simulink library "Renault_Library"  (Simulink 8.2)
settings: Solver=ode3  StartTime=0.0  StopTime=10.0  FixedStep=0.020  RelTol=1e-3
undefined symbols (not defined in the file or in its folder): ABC, AssystemProposal, b, chkval, CoeffA_C, CoeffB_C, CoeffMean_C, ConfTime_C, DiagDelay_C, DividePercent, FailureConfActive_C, FallingEdgeDuration_C, HighLevelThresholdVoltage_C, HighLevelVoltage_C, HighLevelVoltageTunable_C, InitValue_C, InputVoltRangeMax_C, InputVoltRangeMin_C, LowActiveContact_C, LowLevelThresholdVoltage_C, LowLevelVoltage_C, MaxOperatingVoltage_C, MinOperatingVoltage_C, mV2V_conversion, OUT1, OutOfRangeHigh_C, OutOfRangeLow_C, PinSupplySaturationMax, PinSupplySaturationMin, Res1Value_C, Res2Value_C, Res3Value_C, RisingEdgeDuration_C, RSA_CANSignalFailMonitoring, SampleTime_C, service14Table, service19Table, service22Table, service24Table, service28Table, service2ETable, service2FTable, service31Table, service87Table, SizeXStateDID2FTable, SizeXTable14, SizeXTable19, SizeXTable22, SizeXTable24, SizeXTable28, SizeXTable2E, SizeXTable2F, SizeXTable31, SizeXTable87, SizeYStateDID2FTable, SizeYTable14, SizeYTable19, SizeYTable22, SizeYTable24, SizeYTable28 ... (+22)
undefined symbols (not defined in the file): BatteryVoltage_ActivationThreshold_C, FrameStatus_AbsentValue_C, FrameStatus_ConfAbsentValue_C, MemThreshold_C, NAMEOFTHEMODEL_SAMPLE_TIME_CST, NbAbs1_C, NbConfAbs1_C, NbConfPres1_C, NbPres1_C, NOMDUSIGNAL_FaultConfTime_C, NOMDUSIGNAL_FaultRehabTime_C, NOMDUSIGNAL_switchvalue_C, NOMDUSIGNAL_unavailablevalue_C, PeriodFrame1_C, ResetTime_C, WakeUpTime_C
libraries: Renault_Library x112, simulink x75
(part 11 of 32)

== system Renault_Library/Complex blocks/Timers & Counters/RSA_Timer_v01_05 ==
  Init : Inport
      settings: AttributesFormatString=Type : %<OutDataTypeStr>
  Duration : Inport  Port=2
      settings: AttributesFormatString=Type : %<OutDataTypeStr>
  Constant1 : Constant  Tag=AM,Constant,0,1,Value;CMP,Value;  Value=TIM1INI  OutDataTypeStr=Inherit: Inherit via back propagation  SampleTime=-1
  Constant2 : Constant  Tag=AM,Constant,0,1,Value;CMP,Value;  Value=TIM1INI  OutDataTypeStr=Inherit: Inherit via back propagation  SampleTime=-1
  Constant3 : Constant  Tag=AM,Constant,0,1,Value;CMP,Value;  Value=st  OutDataTypeStr=Inherit: Inherit via back propagation  SampleTime=-1
  Constant4 : Constant  Tag=AM,Constant,0,1,Value;CMP,Value;  Value=st  OutDataTypeStr=Inherit: Inherit via back propagation  SampleTime=-1
  Constant5 : Constant  Tag=AM,Constant,0,1,Value;CMP,Value;  Value=1000  OutDataTypeStr=uint16  SampleTime=-1
  Constant6 : Constant  Tag=AM,Constant,0,1,Value;CMP,Value;  OutDataTypeStr=Inherit: Inherit via back propagation  SampleTime=-1
  DataTypeConversion : DataTypeConversion  RndMeth=Zero
  DataTypeConversion1 : DataTypeConversion  OutDataTypeStr=uint32  SaturateOnIntegerOverflow=on  RndMeth=Zero
  DataTypeConversion2 : DataTypeConversion  RndMeth=Zero
  DelayInput1 : UnitDelay  Description=\nStore in Global RAM  InitialCondition=timeoutinit  SampleTime=-1
      settings: AttributesFormatString=IC = %<X0>
  DelayInput2 : UnitDelay  Description=\nStore in Global RAM  InitialCondition=TIM1INI  SampleTime=-1
      settings: AttributesFormatString=IC = %<X0>
  EdgeRising : Subsystem  mask(EdgeRising){vinit=initinit}
      mask prompts: vinit "Initialisation"
      mask help: When a rising edge is used as input, the ouput is equal to one during the fall of the signal. Otherwise the output is zero.
      mask Display:
      | plot([0.2 0.4 0.4 0.6],[0.6 0.6 0.9 0.9]);
      | plot([0.2 0.4 0.4 0.4 0.6],[0.1 0.1 0.4 0.1 0.1])
  LogicalOperator : Logic  OutDataTypeStr=boolean
  Product : Product  SaturateOnIntegerOverflow=on  RndMeth=Zero
  Product1 : Product  SaturateOnIntegerOverflow=on  RndMeth=Zero
  Product2 : Product  Inputs=*/  OutDataTypeStr=Inherit: Inherit via back propagation  SaturateOnIntegerOverflow=on  RndMeth=Zero
  RSA_CompareToZero : Subsystem  Tag=[LIB]  mask(RSA_CompareToZero){relop=~=, LogicOutDataTypeMode=boolean, ZeroCross=on}
      mask prompts: relop "Operator:"; LogicOutDataTypeMode "Output data type mode:"; ZeroCross "Enable zero-crossing detection"
      mask help:
      | RSA_CompareToZero block compares an input signal to zero.
      | Specify how the input is compared to zero with the “Operator” parameter.
      | The “Operator” parameter can have the following values:
      |       1. "==" — Determine whether the input is equal to zero.
      |       2. "~=" — Determine whether the input is not equal to zero.
      |       3. "<"  — Determine whether the input is less than zero.
      |       4. "<=" — Determine whether the input is less than or equal to zero.
      |       5. ">"  — Determine whether the input is greater than zero.
      |       6. ">=" — Determine whether the input is greater than or equal to zero.
      |
      | The output is 0 if the comparison is false, and 1 if it is true.
      |
      | Version : 01.03
      mask init: same as Renault_Library/Complex blocks/Logic & Bit Operations/RSA_CompareToZero
      settings: AttributesFormatString=%<MaskType> : Ver : 01.03\nOperator: %<relop>\nOutput data type mode: %<LogicOutDataTypeMode>\nEnable zero-crossing detection %<ZeroCross>
      mask Help: eval(['open([','''\\F-khepri\shared\DIM-DCMAP-CGMP\66161\Shared_MatLab\Tools_DCMAP\Common_Library_MBDR\Library\Common_Library\Help\RLV_RSA_CompareToZero_Spec_V1.03.pdf''','])'])
      mask TypeOptions relop: == | ~= | < | <= | >= | >
      mask TypeOptions LogicOutDataTypeMode: uint8 | boolean
      mask Display: disp([relop,' 0'])
  RSA_DataTypeDuplicate_v01_03 : Subsystem  Tag=[LIB]  mask(RSA_DataTypeDuplicate){NoIn=2}
      mask prompts: NoIn "No Of Inputs"
      mask help:
      | Force all inputs to have the exact same data type.
      |
      | Version : 01.03
      mask init: same as Renault_Library/Complex blocks/Signal Routing/RSA_DataTypeDuplicate_v01_03
      settings: AttributesFormatString=%<MaskType> : Ver : 01.03\nNo Of Inputs %<NoIn>
      mask Help: eval(['open([','''\\F-khepri\shared\DIM-DCMAP-CGMP\66161\Shared_MatLab\Tools_DCMAP\Common_Library_MBDR\Library\Common_Library\Help\RLV_RSA_DataTypeDuplicate_Spec_V1.03.pdf''','])'])
      mask Display: disp('Same\n DT')
  RelationalOperator1 : RelationalOperator  Operator=>  OutDataTypeStr=boolean  ZeroCross=off  InputSameDT=on
  RelationalOperator2 : RelationalOperator  Operator=<=  OutDataTypeStr=boolean  ZeroCross=off  InputSameDT=on
  Subtract4 : Sum  Inputs=-+  OutDataTypeStr=Inherit: Inherit via back propagation  RndMeth=Zero  SaturateOnIntegerOverflow=on  InputSameDT=on
      settings: AttributesFormatString=SAT = %<SaturateOnIntegerOverflow>
  Sum : Sum  Inputs=+-  OutDataTypeStr=Inherit: Inherit via back propagation  RndMeth=Zero  SaturateOnIntegerOverflow=on  InputSameDT=on
      settings: AttributesFormatString=SAT = %<SaturateOnIntegerOverflow>
  Switch{1..5} (5 alike) : Switch  Tag=GEN,Threshold;AM,Switch,2,1;CMP,Threshold;  Criteria=u2 ~= 0  RndMeth=Zero  SaturateOnIntegerOverflow=on  InputSameDT=on
      settings: AttributesFormatString=Criteria=%<Criteria>
  Timeout : Outport
      settings: AttributesFormatString=Type : %<OutDataTypeStr>\nInit :%<InitialOutput>
  Timer : Outport  Port=2
      settings: AttributesFormatString=Type : %<OutDataTypeStr>\nInit :%<InitialOutput>
  wires:
  Constant2/1 -> Switch3/1
  Switch2/1 -> RelationalOperator1/2, Switch3/3  "X3"
  Constant1/1 -> Switch4/3
  Switch4/1 -> Switch2/3
  RelationalOperator2/1 -> Switch4/2
  DataTypeConversion/1 -> Timer/1
  EdgeRising/1(Out) -> Switch1/2, Switch1/1, [EdgeRising]
  Init/1 -> EdgeRising/1(In)
  LogicalOperator/1 -> Timeout/1
  Switch1/1 -> DelayInput1/1, LogicalOperator/1  "Flag_of_first_initialisation"
  DelayInput1/1 -> Switch1/3
  RelationalOperator1/1 -> Switch3/2, LogicalOperator/2  "X4"
  [Timestep1] -> Sum/2, RelationalOperator2/1
  [EdgeRising] -> Switch2/2
  DelayInput2/1 -> Sum/1, RelationalOperator2/2  "X2"
  Sum/1 -> Switch4/1
  Constant3/1 -> DataTypeConversion1/1, Subtract4/2
  DataTypeConversion1/1 -> DataTypeConversion2/1
  DataTypeConversion2/1 -> Subtract4/1
  RSA_CompareToZero/1(y) -> Switch5/2
  Subtract4/1 -> RSA_CompareToZero/1(u)
  Constant6/1 -> Switch5/3
  Constant5/1 -> Switch5/1
  Switch5/1 -> [tolcons]
  Product/1 -> [Timestep1]
  Constant4/1 -> Product/1, RSA_DataTypeDuplicate_v01_03/1(In1)
  [tolcons] -> Product/2
  Duration/1 -> RSA_DataTypeDuplicate_v01_03/2(In2), Product1/1
  [tolcons] -> Product1/2
  Product1/1 -> Switch2/1
  [Timestep1] -> RelationalOperator1/1
  Switch3/1 -> Product2/1, DelayInput2/1
  [tolcons] -> Product2/2
  Product2/1 -> DataTypeConversion/1

== system Renault_Library/Complex blocks/Timers & Counters/RSA_Timer_v01_06 ==
  Init : Inport
      settings: AttributesFormatString=Type : %<OutDataTypeStr>
  Duration : Inport  Port=2
      settings: AttributesFormatString=Type : %<OutDataTypeStr>
  Constant1 : Constant  Tag=AM,Constant,0,1,Value;CMP,Value;  Value=TIM1INI  OutDataTypeStr=Inherit: Inherit via back propagation  SampleTime=-1
  Constant2 : Constant  Tag=AM,Constant,0,1,Value;CMP,Value;  Value=TIM1INI  OutDataTypeStr=Inherit: Inherit via back propagation  SampleTime=-1
  Constant4 : Constant  Tag=AM,Constant,0,1,Value;CMP,Value;  Value=st  OutDataTypeStr=Inherit: Inherit via back propagation  SampleTime=-1
  Constant5 : Constant  Tag=AM,Constant,0,1,Value;CMP,Value;  Value=1000  OutDataTypeStr=uint16  SampleTime=-1
  Constant6 : Constant  Tag=AM,Constant,0,1,Value;CMP,Value;  OutDataTypeStr=Inherit: Inherit via back propagation  SampleTime=-1
  DataTypeConversion : DataTypeConversion  RndMeth=Zero
  DataTypeConversion1 : DataTypeConversion  OutDataTypeStr=uint32  SaturateOnIntegerOverflow=on  RndMeth=Zero
  DataTypeConversion2 : DataTypeConversion  RndMeth=Zero
  DelayInput1 : UnitDelay  Description=\nStore in Global RAM  InitialCondition=timeoutinit  SampleTime=-1
      settings: AttributesFormatString=IC = %<X0>
  DelayInput2 : UnitDelay  Description=\nStore in Global RAM  InitialCondition=TIM1INI  SampleTime=-1
      settings: AttributesFormatString=IC = %<X0>
  EdgeRising : Subsystem  mask(EdgeRising){vinit=initinit}
      mask prompts: vinit "Initialisation"
      mask help: When a rising edge is used as input, the ouput is equal to one during the fall of the signal. Otherwise the output is zero.
      mask Display:
      | plot([0.2 0.4 0.4 0.6],[0.6 0.6 0.9 0.9]);
      | plot([0.2 0.4 0.4 0.4 0.6],[0.1 0.1 0.4 0.1 0.1])
  LogicalOperator : Logic  OutDataTypeStr=boolean
  Product : Product  SaturateOnIntegerOverflow=on  RndMeth=Zero
  Product1 : Product  SaturateOnIntegerOverflow=on  RndMeth=Zero
  Product2 : Product  Inputs=*/  OutDataTypeStr=Inherit: Inherit via back propagation  SaturateOnIntegerOverflow=on  RndMeth=Zero
  RSA_CompareToZero : Renault_Library/Complex blocks/Logic & Bit Operations/RSA_CompareToZero  Tag=[LIB]  SystemSampleTime=-1  relop=~=  LogicOutDataTypeMode=boolean
      settings: AttributesFormatString=%<MaskType> : Ver : 01.03\nOperator: %<relop>\nOutput data type mode: %<LogicOutDataTypeMode>\nEnable zero-crossing detection %<ZeroCross>
  RSA_DataTypeDuplicate_v01_03 : Renault_Library/Complex blocks/Signal Routing/RSA_DataTypeDuplicate_v01_03  Tag=[LIB]  SystemSampleTime=-1  NoIn=2
      settings: AttributesFormatString=%<MaskType> : Ver : 01.03\nNo Of Inputs %<NoIn>
  RelationalOperator1 : RelationalOperator  Operator=>  OutDataTypeStr=boolean  ZeroCross=off  InputSameDT=on
  RelationalOperator2 : RelationalOperator  Operator=<=  OutDataTypeStr=boolean  ZeroCross=off  InputSameDT=on
  Subtract4 : Sum  Inputs=-+  OutDataTypeStr=Inherit: Inherit via back propagation  RndMeth=Zero  SaturateOnIntegerOverflow=on  InputSameDT=on
      settings: AttributesFormatString=SAT = %<SaturateOnIntegerOverflow>
  Sum : Sum  Inputs=+-  OutDataTypeStr=Inherit: Inherit via back propagation  RndMeth=Zero  SaturateOnIntegerOverflow=on  InputSameDT=on
      settings: AttributesFormatString=SAT = %<SaturateOnIntegerOverflow>
  Switch{1..5} (5 alike) : Switch  Tag=GEN,Threshold;AM,Switch,2,1;CMP,Threshold;  Criteria=u2 ~= 0  RndMeth=Zero  SaturateOnIntegerOverflow=on  InputSameDT=on
      settings: AttributesFormatString=Criteria=%<Criteria>
  Timeout : Outport
      settings: AttributesFormatString=Type : %<OutDataTypeStr>\nInit :%<InitialOutput>
  Timer : Outport  Port=2
      settings: AttributesFormatString=Type : %<OutDataTypeStr>\nInit :%<InitialOutput>
  wires:
  Constant2/1 -> Switch3/1
  Switch2/1 -> RelationalOperator1/2, Switch3/3  "X3"
  Constant1/1 -> Switch4/3
  Switch4/1 -> Switch2/3
  RelationalOperator2/1 -> Switch4/2
  DataTypeConversion/1 -> Timer/1
  EdgeRising/1(Out) -> Switch1/2, Switch1/1, [EdgeRising]
  Init/1 -> EdgeRising/1(In)
  LogicalOperator/1 -> Timeout/1
  Switch1/1 -> DelayInput1/1, LogicalOperator/1  "Flag_of_first_initialisation"
  DelayInput1/1 -> Switch1/3
  RelationalOperator1/1 -> Switch3/2, LogicalOperator/2  "X4"
  [Timestep1] -> Sum/2, RelationalOperator2/1
  [EdgeRising] -> Switch2/2
  DelayInput2/1 -> Sum/1, RelationalOperator2/2  "X2"
  Sum/1 -> Switch4/1
  [st] -> DataTypeConversion1/1, Subtract4/2
  DataTypeConversion1/1 -> DataTypeConversion2/1
  DataTypeConversion2/1 -> Subtract4/1
  RSA_CompareToZero/1 -> Switch5/2
  Subtract4/1 -> RSA_CompareToZero/1
  Constant6/1 -> Switch5/3
  Constant5/1 -> Switch5/1
  Switch5/1 -> [tolcons]
  Product/1 -> [Timestep1]
  Constant4/1 -> RSA_DataTypeDuplicate_v01_03/1, [st], Product/1
  [tolcons] -> Product/2
  Duration/1 -> RSA_DataTypeDuplicate_v01_03/2, Product1/1
  [tolcons] -> Product1/2
  Product1/1 -> Switch2/1
  [Timestep1] -> RelationalOperator1/1
  Switch3/1 -> Product2/1, DelayInput2/1
  [tolcons] -> Product2/2
  Product2/1 -> DataTypeConversion/1

== system Renault_Library/Complex blocks/Timers & Counters/RSA_Timer_v01_07 ==
  note: Flag_of_first_initialisation
  Init : Inport
      settings: AttributesFormatString=Type : %<OutDataTypeStr>
  Duration : Inport  Port=2
      settings: AttributesFormatString=Type : %<OutDataTypeStr>
  Constant1 : Constant  Tag=AM,Constant,0,1,Value;CMP,Value;  Value=TIM1INI  OutDataTypeStr=Inherit: Inherit via back propagation  SampleTime=-1
  Constant2 : Constant  Tag=AM,Constant,0,1,Value;CMP,Value;  Value=TIM1INI  OutDataTypeStr=Inherit: Inherit via back propagation  SampleTime=-1
  Constant3 : Constant  Tag=AM,Constant,0,1,Value;CMP,Value;  Value=st  OutDataTypeStr=SampleDT
  Constant5 : Constant  Value=Boosting_Factor  OutDataTypeStr=Constant_DT
  DelayInput1 : UnitDelay  Description=\nStore in Global RAM  InitialCondition=timeoutinit  SampleTime=-1
      settings: AttributesFormatString=IC = %<X0>
  DelayInput2 : UnitDelay  Description=\nStore in Global RAM  InitialCondition=TIM1INI  SampleTime=-1
      settings: AttributesFormatString=IC = %<X0>
  EdgeRising : Subsystem  mask(EdgeRising){vinit=initinit}
      mask prompts: vinit "Initialisation"
      mask help: When a rising edge is used as input, the ouput is equal to one during the fall of the signal. Otherwise the output is zero.
      mask Display:
      | plot([0.2 0.4 0.4 0.6],[0.6 0.6 0.9 0.9]);
      | plot([0.2 0.4 0.4 0.4 0.6],[0.1 0.1 0.4 0.1 0.1])
  LogicalOperator : Logic  OutDataTypeStr=boolean
  Product2 : Product  Inputs=*/  OutDataTypeStr=Inherit: Inherit via back propagation  SaturateOnIntegerOverflow=on  RndMeth=Zero
  Product3 : Product  OutDataTypeStr=Inherit: Inherit via back propagation  SaturateOnIntegerOverflow=on  RndMeth=Zero
  Product4 : Product  OutDataTypeStr=Inherit: Inherit via back propagation  SaturateOnIntegerOverflow=on  RndMeth=Zero
  RelationalOperator1 : RelationalOperator  Operator=>  OutDataTypeStr=boolean  ZeroCross=off  InputSameDT=on
  RelationalOperator2 : RelationalOperator  Operator=<=  OutDataTypeStr=boolean  ZeroCross=off  InputSameDT=on
  Sum : Sum  Inputs=+-  OutDataTypeStr=Inherit: Inherit via back propagation  RndMeth=Zero  InputSameDT=on
      settings: AttributesFormatString=SAT = %<SaturateOnIntegerOverflow>
  Switch{1..4} (4 alike) : Switch  Tag=GEN,Threshold;AM,Switch,2,1;CMP,Threshold;  Criteria=u2 ~= 0  RndMeth=Zero  SaturateOnIntegerOverflow=on  InputSameDT=on
      settings: AttributesFormatString=Criteria=%<Criteria>
  Timeout : Outport
      settings: AttributesFormatString=Type : %<OutDataTypeStr>\nInit :%<InitialOutput>
  Timer : Outport  Port=2
      settings: AttributesFormatString=Type : %<OutDataTypeStr>\nInit :%<InitialOutput>
  wires:
  Constant2/1 -> Switch3/1
  Switch2/1 -> RelationalOperator1/2, Switch3/3
  Constant1/1 -> Switch4/3
  Switch4/1 -> Switch2/3
  RelationalOperator2/1 -> Switch4/2
  EdgeRising/1(Out) -> Switch1/2, Switch1/1, [EdgeRising]
  Init/1 -> EdgeRising/1(In)
  LogicalOperator/1 -> Timeout/1
  Switch1/1 -> LogicalOperator/1, DelayInput1/1
  DelayInput1/1 -> Switch1/3
  RelationalOperator1/1 -> Switch3/2, LogicalOperator/2
  [Timestep1] -> Sum/2, RelationalOperator2/1
  [EdgeRising] -> Switch2/2
  DelayInput2/1 -> Sum/1, RelationalOperator2/2
  Sum/1 -> Switch4/1
  [Timestep1] -> RelationalOperator1/1
  Switch3/1 -> DelayInput2/1, Product2/1
  [tolcons] -> Product2/2
  Constant5/1 -> [tolcons]
  [tolcons] -> Product3/2
  [tolcons] -> Product4/2
  Product4/1 -> [dly]
  Product3/1 -> [Timestep1]
  Constant3/1 -> Product3/1
  Duration/1 -> Product4/1
  [dly] -> Switch2/1
  Product2/1 -> Timer/1

== system Renault_Library/Complex blocks/Timers & Counters/RSA_TurnOffDelay2 ==
  in : Inport
      settings: AttributesFormatString=Type : %<OutDataTypeStr>
  dly : Inport  Port=2
      settings: AttributesFormatString=Type : %<OutDataTypeStr>
  Constant1 : Constant  Value=INIVAL  OutDataTypeStr=Inherit: Inherit via back propagation  SampleTime=-1
  Constant2 : Constant  Value=Ts  OutDataTypeStr=Inherit: Inherit via back propagation  SampleTime=-1
  Logical_Operator1 : Logic  Operator=OR  OutDataTypeStr=boolean
  Logical_Operator2 : Logic  OutDataTypeStr=boolean
  Logical_Operator3 : Logic  Operator=NOT  OutDataTypeStr=boolean
  Logical_Operator4 : Logic  Operator=NOT  OutDataTypeStr=boolean
  MinMax : MinMax  Function=max  Inputs=2  OutDataTypeStr=Inherit: Inherit via back propagation  RndMeth=Zero  SaturateOnIntegerOverflow=on  InputSameDT=on
  Relational_Operator8 : RelationalOperator  Operator=<=  OutDataTypeStr=boolean  InputSameDT=on
  Sum1 : Sum  Inputs=+-  OutDataTypeStr=Inherit: Inherit via back propagation  RndMeth=Zero  SaturateOnIntegerOverflow=on  InputSameDT=on
      settings: AttributesFormatString=SAT = %<SaturateOnIntegerOverflow>
  Switch1 : Switch  Criteria=u2 ~= 0  RndMeth=Zero  SaturateOnIntegerOverflow=on  InputSameDT=on
      settings: AttributesFormatString=Criteria=%<Criteria>
  Switch2 : Switch  Criteria=u2 ~= 0  RndMeth=Zero  SaturateOnIntegerOverflow=on  InputSameDT=on
      settings: AttributesFormatString=Criteria=%<Criteria>
  UnitDelay : UnitDelay  InitialCondition=false  SampleTime=-1
  Unit_Delay2 : UnitDelay  InitialCondition=INIVAL  SampleTime=-1
  out : Outport
      settings: AttributesFormatString=Type : %<OutDataTypeStr>\nInit :%<InitialOutput>
  ctr : Outport  Port=2
      settings: AttributesFormatString=Type : %<OutDataTypeStr>\nInit :%<InitialOutput>
  wires:
  Constant1/1 -> [INIVAL], Switch1/1
  Constant2/1 -> Sum1/2
  [dly] -> Switch2/1
  [INIVAL] -> Relational_Operator8/2
  Unit_Delay2/1 -> Sum1/1
  Relational_Operator8/1 -> Logical_Operator4/1
  Logical_Operator1/1 -> out/1
  Sum1/1 -> MinMax/1
  MinMax/1 -> Switch2/3
  Switch2/1 -> Switch1/3
  Switch1/1 -> Unit_Delay2/1, ctr/1, Relational_Operator8/1
  UnitDelay/1 -> Logical_Operator2/2
  Logical_Operator2/1 -> Switch2/2
  Logical_Operator3/1 -> Logical_Operator2/1
  Logical_Operator4/1 -> Logical_Operator1/2
  dly/1 -> [dly]
  [INIVAL] -> MinMax/2
  in/1 -> UnitDelay/1, Logical_Operator3/1, Logical_Operator1/1, Switch1/2

== system Renault_Library/Complex blocks/Timers & Counters/RSA_TurnOffDelay2_v01_03 ==
  in : Inport
      settings: AttributesFormatString=Type : %<OutDataTypeStr>
  dly : Inport  Port=2
      settings: AttributesFormatString=Type : %<OutDataTypeStr>
  Constant1 : Constant  Value=INIVAL  OutDataTypeStr=Inherit: Inherit via back propagation  SampleTime=-1
  Constant2 : Constant  Value=Ts  OutDataTypeStr=Inherit: Inherit via back propagation  SampleTime=-1
  Constant3 : Constant  Value=Ts  OutDataTypeStr=Inherit: Inherit via back propagation  SampleTime=-1
  Logical_Operator1 : Logic  Operator=OR  OutDataTypeStr=boolean
  Logical_Operator2 : Logic  OutDataTypeStr=boolean
  Logical_Operator3 : Logic  Operator=NOT  OutDataTypeStr=boolean
  Relational_Operator1 : RelationalOperator  Operator=<=  OutDataTypeStr=boolean  InputSameDT=on
  Relational_Operator8 : RelationalOperator  Operator=>  OutDataTypeStr=boolean  InputSameDT=on
  Sum1 : Sum  Inputs=+-  OutDataTypeStr=Inherit: Inherit via back propagation  RndMeth=Zero  SaturateOnIntegerOverflow=on  InputSameDT=on
      settings: AttributesFormatString=SAT = %<SaturateOnIntegerOverflow>
  Switch1 : Switch  Criteria=u2 ~= 0  RndMeth=Zero  SaturateOnIntegerOverflow=on  InputSameDT=on
      settings: AttributesFormatString=Criteria=%<Criteria>
  Switch2 : Switch  Criteria=u2 ~= 0  RndMeth=Zero  SaturateOnIntegerOverflow=on  InputSameDT=on
      settings: AttributesFormatString=Criteria=%<Criteria>
  Switch3 : Switch  Criteria=u2 ~= 0  RndMeth=Zero  SaturateOnIntegerOverflow=on  InputSameDT=on
      settings: AttributesFormatString=Criteria=%<Criteria>
  UnitDelay : UnitDelay  InitialCondition=false  SampleTime=-1
      settings: AttributesFormatString=IC = %<X0>
  Unit_Delay2 : UnitDelay  InitialCondition=INIVAL  SampleTime=-1
      settings: AttributesFormatString=IC = %<X0>
  out : Outport
      settings: AttributesFormatString=Type : %<OutDataTypeStr>\nInit :%<InitialOutput>
  ctr : Outport  Port=2
      settings: AttributesFormatString=Type : %<OutDataTypeStr>\nInit :%<InitialOutput>
  wires:
  Constant1/1 -> Switch1/1, [INIVAL]
  Constant2/1 -> Sum1/2
  [dly] -> Switch2/1
  [INIVAL] -> Relational_Operator8/2
  Unit_Delay2/1 -> Relational_Operator1/1, Sum1/1
  Logical_Operator1/1 -> out/1
  Switch2/1 -> Switch1/3
  Switch1/1 -> Unit_Delay2/1, ctr/1, Relational_Operator8/1
  UnitDelay/1 -> Logical_Operator2/2
  Logical_Operator2/1 -> Switch2/2
  Logical_Operator3/1 -> Logical_Operator2/1
  dly/1 -> [dly]
  in/1 -> UnitDelay/1, Logical_Operator3/1, Logical_Operator1/1, Switch1/2
  Relational_Operator8/1 -> Logical_Operator1/2
  Switch3/1 -> Switch2/3
  Sum1/1 -> Switch3/3
  Relational_Operator1/1 -> Switch3/2
  [INIVAL] -> Switch3/1
  Constant3/1 -> Relational_Operator1/2

== system Renault_Library/Complex blocks/Timers & Counters/RSA_TurnOffDelay2_v01_05 ==
  in : Inport
      settings: AttributesFormatString=Type : %<OutDataTypeStr>
  dly : Inport  Port=2
      settings: AttributesFormatString=Type : %<OutDataTypeStr>
  Constant1 : Constant  Value=INIVAL  OutDataTypeStr=Inherit: Inherit via back propagation  SampleTime=-1
  Constant4 : Constant  Tag=AM,Constant,0,1,Value;CMP,Value;  Value=Ts  OutDataTypeStr=Inherit: Inherit via back propagation  SampleTime=-1
  Constant5 : Constant  Tag=AM,Constant,0,1,Value;CMP,Value;  Value=1000  OutDataTypeStr=uint16  SampleTime=-1
  Constant6 : Constant  Tag=AM,Constant,0,1,Value;CMP,Value;  OutDataTypeStr=Inherit: Inherit via back propagation  SampleTime=-1
  DataTypeConversion1 : DataTypeConversion  OutDataTypeStr=uint32  SaturateOnIntegerOverflow=on  RndMeth=Zero
  DataTypeConversion2 : DataTypeConversion  RndMeth=Zero
  DataTypeConversion3 : DataTypeConversion  RndMeth=Zero
  Logical_Operator1 : Logic  Operator=OR  OutDataTypeStr=boolean
  Logical_Operator2 : Logic  OutDataTypeStr=boolean
  Logical_Operator3 : Logic  Operator=NOT  OutDataTypeStr=boolean
  Product : Product  SaturateOnIntegerOverflow=on  RndMeth=Zero
  Product1 : Product  SaturateOnIntegerOverflow=on  RndMeth=Zero
  Product2 : Product  Inputs=*/  SaturateOnIntegerOverflow=on  RndMeth=Zero
  RSA_CompareToZero : Renault_Library/Complex blocks/Logic & Bit Operations/RSA_CompareToZero  Tag=[LIB]  SystemSampleTime=-1  relop===  LogicOutDataTypeMode=boolean
      settings: AttributesFormatString=%<MaskType> : Ver : 01.03\nOperator: %<relop>\nOutput data type mode: %<LogicOutDataTypeMode>\nEnable zero-crossing detection %<ZeroCross>
  RSA_DataTypeDuplicate_v01_03 : Renault_Library/Complex blocks/Signal Routing/RSA_DataTypeDuplicate_v01_03  Tag=[LIB]  SystemSampleTime=-1  NoIn=2
      settings: AttributesFormatString=%<MaskType> : Ver : 01.03\nNo Of Inputs %<NoIn>
  Relational_Operator1 : RelationalOperator  Operator=<=  OutDataTypeStr=boolean  InputSameDT=on
  Relational_Operator8 : RelationalOperator  OutDataTypeStr=boolean  InputSameDT=on
  Subtract4 : Sum  Inputs=-+  OutDataTypeStr=Inherit: Inherit via back propagation  RndMeth=Zero  SaturateOnIntegerOverflow=on  InputSameDT=on
      settings: AttributesFormatString=SAT = %<SaturateOnIntegerOverflow>
  Sum1 : Sum  Inputs=+-  OutDataTypeStr=Inherit: Inherit via back propagation  RndMeth=Zero  SaturateOnIntegerOverflow=on  InputSameDT=on
      settings: AttributesFormatString=SAT = %<SaturateOnIntegerOverflow>
  Switch1 : Switch  Criteria=u2 ~= 0  RndMeth=Zero  SaturateOnIntegerOverflow=on  InputSameDT=on
      settings: AttributesFormatString=Criteria=%<Criteria>
  Switch2 : Switch  Criteria=u2 ~= 0  RndMeth=Zero  SaturateOnIntegerOverflow=on  InputSameDT=on
      settings: AttributesFormatString=Criteria=%<Criteria>
  Switch3 : Switch  Criteria=u2 ~= 0  RndMeth=Zero  SaturateOnIntegerOverflow=on  InputSameDT=on
      settings: AttributesFormatString=Criteria=%<Criteria>
  Switch5 : Switch  Tag=GEN,Threshold;AM,Switch,2,1;CMP,Threshold;  Criteria=u2 ~= 0  RndMeth=Zero  SaturateOnIntegerOverflow=on  InputSameDT=on
      settings: AttributesFormatString=Criteria=%<Criteria>
  UnitDelay : UnitDelay  InitialCondition=false  SampleTime=-1
      settings: AttributesFormatString=IC = %<X0>
  Unit_Delay2 : UnitDelay  InitialCondition=INIVAL  SampleTime=-1
      settings: AttributesFormatString=IC = %<X0>
  out : Outport
      settings: AttributesFormatString=Type : %<OutDataTypeStr>\nInit :%<InitialOutput>
  ctr : Outport  Port=2
      settings: AttributesFormatString=Type : %<OutDataTypeStr>\nInit :%<InitialOutput>
  wires:
  Constant1/1 -> Switch1/1, [INIVAL]
  [Timestep1] -> Sum1/2
  [dly] -> Switch2/1
  Unit_Delay2/1 -> Relational_Operator1/1, Sum1/1
  Logical_Operator1/1 -> out/1
  Switch2/1 -> Switch1/3
  Switch1/1 -> Product2/1, Unit_Delay2/1, Relational_Operator8/1
  UnitDelay/1 -> Logical_Operator2/2
  Logical_Operator2/1 -> Switch2/2
  Logical_Operator3/1 -> Logical_Operator2/1
  in/1 -> UnitDelay/1, Logical_Operator3/1, Logical_Operator1/1, Switch1/2
  Relational_Operator8/1 -> Logical_Operator1/2
  Switch3/1 -> Switch2/3
  Sum1/1 -> Switch3/3
  Relational_Operator1/1 -> Switch3/2
  [INIVAL] -> Switch3/1
  [Timestep1] -> Relational_Operator1/2
  DataTypeConversion1/1 -> DataTypeConversion2/1
  DataTypeConversion2/1 -> Subtract4/1
  RSA_CompareToZero/1 -> Switch5/2
  Subtract4/1 -> RSA_CompareToZero/1
  Constant6/1 -> Switch5/3
  Constant5/1 -> Switch5/1
  Switch5/1 -> [tolcons]
  Product/1 -> [Timestep1]
  [tolcons] -> Product/2
  Constant4/1 -> RSA_DataTypeDuplicate_v01_03/2, [Ts], Product/1
  [Ts] -> Subtract4/2, DataTypeConversion1/1
  [tolcons] -> Product1/2
  dly/1 -> RSA_DataTypeDuplicate_v01_03/1, Product1/1
  Product1/1 -> [dly]
  Product2/1 -> DataTypeConversion3/1
  [tolcons] -> Product2/2
  [Timestep1] -> Relational_Operator8/2
  DataTypeConversion3/1 -> ctr/1

== system Renault_Library/Complex blocks/Timers & Counters/RSA_TurnOffDelay2_v01_06 ==
  delay_flag : Inport
      settings: AttributesFormatString=Type : %<OutDataTypeStr>
  dly : Inport  Port=2
      settings: AttributesFormatString=Type : %<OutDataTypeStr>
  Constant1 : Constant  Value=INIVAL  OutDataTypeStr=Inherit: Inherit via back propagation  SampleTime=-1
  Constant2 : Constant  Value=Ts  OutDataTypeStr=SampleDT
  Constant3 : Constant  Value=Boosting_Factor  OutDataTypeStr=Constant_DT
  Logical_Operator1 : Logic  Operator=OR  OutDataTypeStr=boolean
  Product2 : Product  Inputs=*/  OutDataTypeStr=Inherit: Inherit via back propagation  SaturateOnIntegerOverflow=on  RndMeth=Zero
  Product3 : Product  OutDataTypeStr=Inherit: Inherit via back propagation  SaturateOnIntegerOverflow=on  RndMeth=Zero
  Product4 : Product  OutDataTypeStr=Inherit: Inherit via back propagation  SaturateOnIntegerOverflow=on  RndMeth=Zero
  Relational_Operator1 : RelationalOperator  Operator=<=  OutDataTypeStr=boolean  InputSameDT=on
  Relational_Operator8 : RelationalOperator  OutDataTypeStr=boolean  InputSameDT=on
  Sum1 : Sum  Inputs=+-  OutDataTypeStr=Inherit: Inherit via back propagation  RndMeth=Zero  SaturateOnIntegerOverflow=on  InputSameDT=on
      settings: AttributesFormatString=SAT = %<SaturateOnIntegerOverflow>
  Switch1 : Switch  Criteria=u2 ~= 0  RndMeth=Zero  SaturateOnIntegerOverflow=on  InputSameDT=on
      settings: AttributesFormatString=Criteria=%<Criteria>
  Switch2 : Switch  Criteria=u2 ~= 0  RndMeth=Zero  SaturateOnIntegerOverflow=on  InputSameDT=on
      settings: AttributesFormatString=Criteria=%<Criteria>
  Switch3 : Switch  Criteria=u2 ~= 0  RndMeth=Zero  SaturateOnIntegerOverflow=on  InputSameDT=on
      settings: AttributesFormatString=Criteria=%<Criteria>
  UnitDelay : UnitDelay  InitialCondition=false  SampleTime=-1
      settings: AttributesFormatString=IC = %<X0>
  Unit_Delay2 : UnitDelay  InitialCondition=INIVAL  SampleTime=-1
      settings: AttributesFormatString=IC = %<X0>
  delay_out : Outport
      settings: AttributesFormatString=Type : %<OutDataTypeStr>\nInit :%<InitialOutput>
  ctr : Outport  Port=2
      settings: AttributesFormatString=Type : %<OutDataTypeStr>\nInit :%<InitialOutput>
  wires:
  [tolcons] -> Product3/2
  dly/1 -> Product4/1
  Constant2/1 -> Product3/1
  Product3/1 -> [Timestep]
  Product4/1 -> [dly]
  [tolcons] -> Product4/2
  Constant3/1 -> [tolcons]
  Constant1/1 -> Switch1/1, [INIVAL]
  [Timestep] -> Sum1/2
  [dly] -> Switch2/1
  Unit_Delay2/1 -> Relational_Operator1/1, Sum1/1
  Logical_Operator1/1 -> delay_out/1
  Switch2/1 -> Switch1/3
  Switch1/1 -> Product2/1, Unit_Delay2/1, Relational_Operator8/1
  UnitDelay/1 -> Switch2/2
  delay_flag/1 -> UnitDelay/1, Logical_Operator1/1, Switch1/2
  Relational_Operator8/1 -> Logical_Operator1/2
  Switch3/1 -> Switch2/3
  Sum1/1 -> Switch3/3
  Relational_Operator1/1 -> Switch3/2
  [INIVAL] -> Switch3/1
  [Timestep] -> Relational_Operator1/2
  [tolcons] -> Product2/2
  [Timestep] -> Relational_Operator8/2
  Product2/1 -> ctr/1

== system Renault_Library/Complex blocks/Timers & Counters/RSA_TurnOffDelay_v01_00 ==
  Delaytime : Inport  Tag=AM,In,0,1,Name,C;CMP,Port,PortWidth,SampleTime;
      settings: AttributesFormatString=Type : %<OutDataTypeStr>
  Signal : Inport  Tag=AM,In,0,1,Name,C;CMP,Port,PortWidth,SampleTime;  Port=2
      settings: AttributesFormatString=Type : %<OutDataTypeStr>
  Timestep : Inport  Tag=AM,In,0,1,Name,C;CMP,Port,PortWidth,SampleTime;  Port=3
      settings: AttributesFormatString=Type : %<OutDataTypeStr>
  Constant1 : Constant  Tag=AM,Constant,0,1,Value;CMP,Value;  Value=TIM1INI  OutDataTypeStr=Inherit: Inherit via back propagation
  Constant2 : Constant  Value=CONS_ONE  OutDataTypeStr=Inherit: Inherit via back propagation
  Constant3 : Constant  Value=CONS_ZERO  OutDataTypeStr=Inherit: Inherit via back propagation
  Constant6 : Constant  Value=CONS_ZERO  OutDataTypeStr=Inherit: Inherit via back propagation
  Constant7 : Constant  Value=BOOSTING_FLP  OutDataTypeStr=uint32
  Constant8 : Constant  Value=BOOSTING_FXP  OutDataTypeStr=Inherit: Inherit via back propagation
  DataTypeConversion2 : DataTypeConversion  RndMeth=Zero
  DelayInput1 : UnitDelay  Description=\nStore in Global RAM  InitialCondition=timeoutinit  SampleTime=-1
      settings: AttributesFormatString=IC = %<X0>
  DelayInput2 : UnitDelay  Description=\nStore in Global RAM  InitialCondition=TIM1INI  SampleTime=-1
      settings: AttributesFormatString=IC = %<X0>
  FallingEdge : Subsystem
  LogicalOperator : Logic  Tag=AM,Std,Inputs,1;CMP,Operator,Inputs;  Operator=OR  OutDataTypeStr=boolean
  LogicalOperator1 : Logic  Tag=AM,Std,Inputs,1;CMP,Operator,Inputs;  Operator=OR  OutDataTypeStr=boolean
  Mux : Mux  Inputs=2
  Product : Product  OutDataTypeStr=Inherit: Inherit via back propagation  SaturateOnIntegerOverflow=on  RndMeth=Zero
  Product1 : Product  OutDataTypeStr=Inherit: Inherit via back propagation  SaturateOnIntegerOverflow=on  RndMeth=Zero
  Product2 : Product  OutDataTypeStr=uint32  SaturateOnIntegerOverflow=on  RndMeth=Zero
  Product3 : Product  Inputs=*/  OutDataTypeStr=Inherit: Inherit via back propagation  SaturateOnIntegerOverflow=on  RndMeth=Zero
  RelationalOperator1 : RelationalOperator  OutDataTypeStr=boolean  ZeroCross=off  InputSameDT=on
  RelationalOperator2 : RelationalOperator  Operator=<=  OutDataTypeStr=boolean  ZeroCross=off  InputSameDT=on
  RelationalOperator3 : RelationalOperator  Operator=>  OutDataTypeStr=boolean  ZeroCross=off  InputSameDT=on
  Subtract4 : Sum  Inputs=-+  OutDataTypeStr=Inherit: Inherit via back propagation  RndMeth=Zero  InputSameDT=on
      settings: AttributesFormatString=SAT = %<SaturateOnIntegerOverflow>
  Sum : Sum  Inputs=+-  OutDataTypeStr=Inherit: Inherit via back propagation  RndMeth=Zero  InputSameDT=on
      settings: AttributesFormatString=SAT = %<SaturateOnIntegerOverflow>
  Switch{1..2,4..5} (4 alike) : Switch  Tag=GEN,Threshold;AM,Switch,2,1;CMP,Threshold;  Criteria=u2 ~= 0  RndMeth=Zero  SaturateOnIntegerOverflow=on  InputSameDT=on
      settings: AttributesFormatString=Criteria=%<Criteria>
  Terminator : Terminator
  Terminator1 : Terminator
  Terminator2 : Terminator
  Out : Outport  Tag=AM,Out,1,0,Name,NC;CMP,Port,OutputWhenDisabled,InitialOutput;
      settings: AttributesFormatString=Type : %<OutDataTypeStr>\nInit :%<InitialOutput>
  ctr : Outport  Port=2
      settings: AttributesFormatString=Type : %<OutDataTypeStr>\nInit :%<InitialOutput>
  wires:
  [InitOut] -> LogicalOperator/2
  RelationalOperator1/1 -> [InitOut_Cond]
  Switch2/1 -> RelationalOperator1/1, DelayInput2/1, Product3/1
  [Timestep1] -> RelationalOperator1/2
  [tolcons] -> Product3/2
  Product3/1 -> ctr/1
  Constant6/1 -> RelationalOperator3/2
  Constant2/1 -> Product2/1
  Switch5/1 -> [tolcons]
  Constant7/1 -> Switch5/1
  Constant8/1 -> Switch5/3
  Subtract4/1 -> RelationalOperator3/1
  RelationalOperator3/1 -> Switch5/2
  DataTypeConversion2/1 -> Subtract4/1
  Product2/1 -> DataTypeConversion2/1
  Sum/1 -> Switch4/1
  Product1/1 -> Switch2/1
  [tolcons] -> Product1/2
  Delaytime/1 -> Mux/2, Product1/1
  [tolcons] -> Product/2
  Product/1 -> [Timestep1]
  LogicalOperator/1 -> Out/1
  Signal/1 -> LogicalOperator/1, FallingEdge/1(In)  "Signal"
  Constant1/1 -> Switch4/3
  DelayInput2/1 -> Sum/1, RelationalOperator2/2
  RelationalOperator2/1 -> Switch4/2
  Switch4/1 -> Switch2/3
  FallingEdge/1(Out) -> [FallingEdge]
  [FallingEdge] -> Switch2/2
  [Timestep1] -> Sum/2, RelationalOperator2/1
  Mux/1 -> Terminator/1
  Timestep/1 -> Mux/1, Product/1, [Timestep]
  [Timestep] -> Product2/2, Subtract4/2
  DelayInput1/1 -> Switch1/3
  Constant3/1 -> Switch1/1
  [FallingEdge] -> Switch1/2
  [InitOut_Cond] -> LogicalOperator1/2
  Switch1/1 -> LogicalOperator1/1, DelayInput1/1
  LogicalOperator1/1 -> [InitOut]
  [InitOut_Cond] -> Terminator1/1
  [InitOut] -> Terminator2/1

== system Renault_Library/Complex blocks/Timers & Counters/RSA_TurnOnDelay ==
  Delaytime : Inport  Tag=AM,In,0,1,Name,C;CMP,Port,PortWidth,SampleTime;
      settings: AttributesFormatString=Type : %<OutDataTypeStr>
  Signal : Inport  Tag=AM,In,0,1,Name,C;CMP,Port,PortWidth,SampleTime;  Port=2
      settings: AttributesFormatString=Type : %<OutDataTypeStr>
  Timestep : Inport  Tag=AM,In,0,1,Name,C;CMP,Port,PortWidth,SampleTime;  Port=3
      settings: AttributesFormatString=Type : %<OutDataTypeStr>
  LogicalOperator : Logic  Tag=AM,Std,Inputs,1;CMP,Operator,Inputs;  OutDataTypeStr=boolean
  RSA_Timer2 : Renault_Library/Complex blocks/Timers & Counters/RSA_Timer1  Tag=[LIB]  timeoutinit=timeoutinit  initinit=initinit
      settings: AttributesFormatString=%<MaskType> : Ver : 01.03\nTimeout output initialisation=%<timeoutinit>\nInit input initialisation=%<initinit>
  Terminator : Terminator
  Out : Outport  Tag=AM,Out,1,0,Name,NC;CMP,Port,OutputWhenDisabled,InitialOutput;
      settings: AttributesFormatString=Type : %<OutDataTypeStr>\nInit :%<InitialOutput>
  wires:
  Timestep/1 -> RSA_Timer2/3
  RSA_Timer2/1 -> LogicalOperator/2  "Timeout"
  LogicalOperator/1 -> Out/1
  Signal/1 -> LogicalOperator/1, RSA_Timer2/1  "Signal"
  RSA_Timer2/2 -> Terminator/1  "Timer"
  Delaytime/1 -> RSA_Timer2/2

== system Renault_Library/Complex blocks/Timers & Counters/RSA_TurnOnDelay2 ==
  in : Inport
      settings: AttributesFormatString=Type : %<OutDataTypeStr>
  dly : Inport  Port=2
      settings: AttributesFormatString=Type : %<OutDataTypeStr>
  Constant2 : Constant  Value=Ts  OutDataTypeStr=Inherit: Inherit via back propagation  SampleTime=-1
  Constant5 : Constant  Value=INIVAL  OutDataTypeStr=Inherit: Inherit via back propagation  SampleTime=-1
  Logical_Operator : Logic  Operator=NOT  OutDataTypeStr=boolean
  Logical_Operator1 : Logic  OutDataTypeStr=boolean
  Logical_Operator2 : Logic  OutDataTypeStr=boolean
  Logical_Operator6 : Logic  Operator=NOT  OutDataTypeStr=boolean
  MinMax : MinMax  Function=max  Inputs=2  OutDataTypeStr=Inherit: Inherit via back propagation  RndMeth=Zero  SaturateOnIntegerOverflow=on  InputSameDT=on
  Relational_Operator8 : RelationalOperator  Operator=<=  OutDataTypeStr=boolean  InputSameDT=on
  Sum1 : Sum  Inputs=+-  OutDataTypeStr=Inherit: Inherit via back propagation  RndMeth=Zero  SaturateOnIntegerOverflow=on  InputSameDT=on
      settings: AttributesFormatString=SAT = %<SaturateOnIntegerOverflow>
  Switch1 : Switch  Criteria=u2 ~= 0  RndMeth=Zero  SaturateOnIntegerOverflow=on  InputSameDT=on
      settings: AttributesFormatString=Criteria=%<Criteria>
  Switch2 : Switch  Criteria=u2 ~= 0  RndMeth=Zero  SaturateOnIntegerOverflow=on  InputSameDT=on
      settings: AttributesFormatString=Criteria=%<Criteria>
  UnitDelay : UnitDelay  InitialCondition=false  SampleTime=-1
  Unit_Delay2 : UnitDelay  InitialCondition=INIVAL  SampleTime=-1
  out : Outport  InitialOutput=[OUT1]
      settings: AttributesFormatString=Type : %<OutDataTypeStr>\nInit :%<InitialOutput>
  ctr : Outport  Port=2  InitialOutput=[OUT1]
      settings: AttributesFormatString=Type : %<OutDataTypeStr>\nInit :%<InitialOutput>
  wires:
  Logical_Operator/1 -> Switch1/2
  Constant2/1 -> Sum1/2
  [INIVAL] -> Relational_Operator8/2
  [INIVAL] -> MinMax/2
  Unit_Delay2/1 -> Sum1/1
  Relational_Operator8/1 -> Logical_Operator1/2
  Logical_Operator1/1 -> out/1
  Sum1/1 -> MinMax/1
  Switch2/1 -> Switch1/3
  Switch1/1 -> Relational_Operator8/1, Unit_Delay2/1, ctr/1
  UnitDelay/1 -> Logical_Operator6/1
  Logical_Operator6/1 -> Logical_Operator2/2
  Logical_Operator2/1 -> Switch2/2
  [dly] -> Switch2/1
  dly/1 -> [dly]
  MinMax/1 -> Switch2/3
  in/1 -> Logical_Operator/1, Logical_Operator2/1, UnitDelay/1, Logical_Operator1/1
  Constant5/1 -> Switch1/1, [INIVAL]

== system Renault_Library/Complex blocks/Timers & Counters/RSA_TurnOnDelay2_v01_03 ==
  in : Inport
      settings: AttributesFormatString=Type : %<OutDataTypeStr>
  dly : Inport  Port=2
      settings: AttributesFormatString=Type : %<OutDataTypeStr>
  Constant1 : Constant  Value=Ts  OutDataTypeStr=Inherit: Inherit via back propagation  SampleTime=-1
  Constant2 : Constant  Value=Ts  OutDataTypeStr=Inherit: Inherit via back propagation  SampleTime=-1
  Constant5 : Constant  Value=INIVAL  OutDataTypeStr=Inherit: Inherit via back propagation  SampleTime=-1
  Logical_Operator : Logic  Operator=NOT  OutDataTypeStr=boolean
  Logical_Operator1 : Logic  OutDataTypeStr=boolean
  Logical_Operator2 : Logic  OutDataTypeStr=boolean
  Logical_Operator6 : Logic  Operator=NOT  OutDataTypeStr=boolean
  Relational_Operator1 : RelationalOperator  Operator=<=  OutDataTypeStr=boolean  InputSameDT=on
  Relational_Operator8 : RelationalOperator  Operator=<=  OutDataTypeStr=boolean  InputSameDT=on
  Sum1 : Sum  Inputs=+-  OutDataTypeStr=Inherit: Inherit via back propagation  RndMeth=Zero  SaturateOnIntegerOverflow=on  InputSameDT=on
      settings: AttributesFormatString=SAT = %<SaturateOnIntegerOverflow>
  Switch1 : Switch  Criteria=u2 ~= 0  RndMeth=Zero  SaturateOnIntegerOverflow=on  InputSameDT=on
      settings: AttributesFormatString=Criteria=%<Criteria>
  Switch2 : Switch  Criteria=u2 ~= 0  RndMeth=Zero  SaturateOnIntegerOverflow=on  InputSameDT=on
      settings: AttributesFormatString=Criteria=%<Criteria>
  Switch3 : Switch  Criteria=u2 ~= 0  RndMeth=Zero  SaturateOnIntegerOverflow=on  InputSameDT=on
      settings: AttributesFormatString=Criteria=%<Criteria>
  UnitDelay : UnitDelay  InitialCondition=false  SampleTime=-1
      settings: AttributesFormatString=IC = %<X0>
  Unit_Delay2 : UnitDelay  InitialCondition=INIVAL  SampleTime=-1
      settings: AttributesFormatString=IC = %<X0>
  out : Outport
      settings: AttributesFormatString=Type : %<OutDataTypeStr>\nInit :%<InitialOutput>
  ctr : Outport  Port=2
      settings: AttributesFormatString=Type : %<OutDataTypeStr>\nInit :%<InitialOutput>
  wires:
  Constant1/1 -> Relational_Operator1/2
  Constant5/1 -> [INIVAL], Switch1/1
  in/1 -> Logical_Operator1/1, UnitDelay/1, Logical_Operator2/1, Logical_Operator/1
  dly/1 -> [dly]
  [dly] -> Switch2/1
  Logical_Operator2/1 -> Switch2/2
  Logical_Operator6/1 -> Logical_Operator2/2
  UnitDelay/1 -> Logical_Operator6/1
  Switch1/1 -> Relational_Operator8/1, ctr/1, Unit_Delay2/1
  Switch2/1 -> Switch1/3
  Logical_Operator1/1 -> out/1
  Relational_Operator8/1 -> Logical_Operator1/2
  [INIVAL] -> Relational_Operator8/2
  Logical_Operator/1 -> Switch1/2
  Unit_Delay2/1 -> Sum1/1, Relational_Operator1/1
  Relational_Operator1/1 -> Switch3/2
  Sum1/1 -> Switch3/3
  Constant2/1 -> Sum1/2
  Switch3/1 -> Switch2/3
  [INIVAL] -> Switch3/1

== system Renault_Library/Complex blocks/Timers & Counters/RSA_TurnOnDelay2_v01_04 ==
  in : Inport
      settings: AttributesFormatString=Type : %<OutDataTypeStr>
  dly : Inport  Port=2
      settings: AttributesFormatString=Type : %<OutDataTypeStr>
  Constant1 : Constant  Value=Ts  OutDataTypeStr=Inherit: Inherit via back propagation  SampleTime=-1
  Constant3 : Constant  Tag=AM,Constant,0,1,Value;CMP,Value;  Value=Ts  OutDataTypeStr=Inherit: Inherit via back propagation  SampleTime=-1
  Constant4 : Constant  Tag=AM,Constant,0,1,Value;CMP,Value;  Value=1000  OutDataTypeStr=uint16  SampleTime=-1
  Constant5 : Constant  Value=INIVAL  OutDataTypeStr=Inherit: Inherit via back propagation  SampleTime=-1
  Constant6 : Constant  Tag=AM,Constant,0,1,Value;CMP,Value;  OutDataTypeStr=Inherit: Inherit via back propagation  SampleTime=-1
  DataTypeConversion1 : DataTypeConversion  OutDataTypeStr=uint32  SaturateOnIntegerOverflow=on  RndMeth=Zero
  DataTypeConversion2 : DataTypeConversion  RndMeth=Zero
  DataTypeConversion3 : DataTypeConversion  RndMeth=Zero
  Logical_Operator : Logic  Operator=NOT  OutDataTypeStr=boolean
  Logical_Operator1 : Logic  OutDataTypeStr=boolean
  Logical_Operator2 : Logic  OutDataTypeStr=boolean
  Logical_Operator6 : Logic  Operator=NOT  OutDataTypeStr=boolean
  Product : Product  SaturateOnIntegerOverflow=on  RndMeth=Zero
  Product1 : Product  SaturateOnIntegerOverflow=on  RndMeth=Zero
  Product2 : Product  Inputs=*/  SaturateOnIntegerOverflow=on  RndMeth=Zero
  RSA_CompareToZero : Subsystem  Tag=[LIB]  mask(RSA_CompareToZero){relop=~=, LogicOutDataTypeMode=boolean, ZeroCross=on}
      mask prompts: relop "Operator:"; LogicOutDataTypeMode "Output data type mode:"; ZeroCross "Enable zero-crossing detection"
      mask help:
      | RSA_CompareToZero block compares an input signal to zero.
      | Specify how the input is compared to zero with the “Operator” parameter.
      | The “Operator” parameter can have the following values:
      |       1. "==" — Determine whether the input is equal to zero.
      |       2. "~=" — Determine whether the input is not equal to zero.
      |       3. "<"  — Determine whether the input is less than zero.
      |       4. "<=" — Determine whether the input is less than or equal to zero.
      |       5. ">"  — Determine whether the input is greater than zero.
      |       6. ">=" — Determine whether the input is greater than or equal to zero.
      |
      | The output is 0 if the comparison is false, and 1 if it is true.
      |
      | Version : 01.03
      mask init: same as Renault_Library/Complex blocks/Logic & Bit Operations/RSA_CompareToZero
      settings: AttributesFormatString=%<MaskType> : Ver : 01.03\nOperator: %<relop>\nOutput data type mode: %<LogicOutDataTypeMode>\nEnable zero-crossing detection %<ZeroCross>
      mask Help: eval(['open([','''\\F-khepri\shared\DIM-DCMAP-CGMP\66161\Shared_MatLab\Tools_DCMAP\Common_Library_MBDR\Library\Common_Library\Help\RLV_RSA_CompareToZero_Spec_V1.03.pdf''','])'])
      mask TypeOptions relop: == | ~= | < | <= | >= | >
      mask TypeOptions LogicOutDataTypeMode: uint8 | boolean
      mask Display: disp([relop,' 0'])
  RSA_DataTypeDuplicate_v01_03 : Subsystem  Tag=[LIB]  mask(RSA_DataTypeDuplicate){NoIn=2}
      mask prompts: NoIn "No Of Inputs"
      mask help:
      | Force all inputs to have the exact same data type.
      |
      | Version : 01.03
      mask init: same as Renault_Library/Complex blocks/Signal Routing/RSA_DataTypeDuplicate_v01_03
      settings: AttributesFormatString=%<MaskType> : Ver : 01.03\nNo Of Inputs %<NoIn>
      mask Help: eval(['open([','''\\F-khepri\shared\DIM-DCMAP-CGMP\66161\Shared_MatLab\Tools_DCMAP\Common_Library_MBDR\Library\Common_Library\Help\RLV_RSA_DataTypeDuplicate_Spec_V1.03.pdf''','])'])
      mask Display: disp('Same\n DT')
  Relational_Operator1 : RelationalOperator  Operator=<=  OutDataTypeStr=boolean  InputSameDT=on
  Relational_Operator8 : RelationalOperator  Operator=>  OutDataTypeStr=boolean  InputSameDT=on
  Subtract4 : Sum  Inputs=-+  OutDataTypeStr=Inherit: Inherit via back propagation  RndMeth=Zero  SaturateOnIntegerOverflow=on  InputSameDT=on
      settings: AttributesFormatString=SAT = %<SaturateOnIntegerOverflow>
  Sum1 : Sum  Inputs=+-  OutDataTypeStr=Inherit: Inherit via back propagation  RndMeth=Zero  SaturateOnIntegerOverflow=on  InputSameDT=on
      settings: AttributesFormatString=SAT = %<SaturateOnIntegerOverflow>
  Switch1 : Switch  Criteria=u2 ~= 0  RndMeth=Zero  SaturateOnIntegerOverflow=on  InputSameDT=on
      settings: AttributesFormatString=Criteria=%<Criteria>
  Switch2 : Switch  Criteria=u2 ~= 0  RndMeth=Zero  SaturateOnIntegerOverflow=on  InputSameDT=on
      settings: AttributesFormatString=Criteria=%<Criteria>
  Switch3 : Switch  Criteria=u2 ~= 0  RndMeth=Zero  SaturateOnIntegerOverflow=on  InputSameDT=on
      settings: AttributesFormatString=Criteria=%<Criteria>
  Switch5 : Switch  Tag=GEN,Threshold;AM,Switch,2,1;CMP,Threshold;  Criteria=u2 ~= 0  RndMeth=Zero  SaturateOnIntegerOverflow=on  InputSameDT=on
      settings: AttributesFormatString=Criteria=%<Criteria>
  UnitDelay : UnitDelay  InitialCondition=false  SampleTime=-1
      settings: AttributesFormatString=IC = %<X0>
  Unit_Delay2 : UnitDelay  InitialCondition=INIVAL  SampleTime=-1
      settings: AttributesFormatString=IC = %<X0>
  out : Outport
      settings: AttributesFormatString=Type : %<OutDataTypeStr>\nInit :%<InitialOutput>
  ctr : Outport  Port=2
      settings: AttributesFormatString=Type : %<OutDataTypeStr>\nInit :%<InitialOutput>
  wires:
  Constant5/1 -> [INIVAL], Switch1/1
  in/1 -> Logical_Operator1/1, UnitDelay/1, Logical_Operator2/1, Logical_Operator/1
  [dly] -> Switch2/1
  Logical_Operator2/1 -> Switch2/2
  Logical_Operator6/1 -> Logical_Operator2/2
  UnitDelay/1 -> Logical_Operator6/1
  Switch1/1 -> Product2/1, Unit_Delay2/1, Relational_Operator8/2
  Switch2/1 -> Switch1/3
  Logical_Operator1/1 -> out/1
  Relational_Operator8/1 -> Logical_Operator1/2
  Logical_Operator/1 -> Switch1/2
  Unit_Delay2/1 -> Sum1/1, Relational_Operator1/1
  Relational_Operator1/1 -> Switch3/2
  Sum1/1 -> Switch3/3
  [Timestep] -> Sum1/2
  Switch3/1 -> Switch2/3
  [INIVAL] -> Switch3/1
  Constant3/1 -> DataTypeConversion1/1, Subtract4/2
  DataTypeConversion1/1 -> DataTypeConversion2/1
  DataTypeConversion2/1 -> Subtract4/1
  RSA_CompareToZero/1(y) -> Switch5/2
  Subtract4/1 -> RSA_CompareToZero/1(u)
  Constant6/1 -> Switch5/3
  Constant4/1 -> Switch5/1
  Switch5/1 -> [tolcons]
  Product/1 -> [Timestep]
  Constant1/1 -> RSA_DataTypeDuplicate_v01_03/2(In2), Product/1
  [tolcons] -> Product/2
  [Timestep] -> Relational_Operator1/2
  [Timestep] -> Relational_Operator8/1
  Product1/1 -> [dly]
  [tolcons] -> Product1/1
  dly/1 -> RSA_DataTypeDuplicate_v01_03/1(In1), Product1/2
  DataTypeConversion3/1 -> ctr/1
  Product2/1 -> DataTypeConversion3/1
  [tolcons] -> Product2/2
